simulink model "ClimaMgrForVentAndFlrDistbn"  (R2022b)
settings: Solver=FixedStepDiscrete  StartTime=0.0  StopTime=10.0  FixedStep=1  RelTol=1e-3
(part 2 of 2)

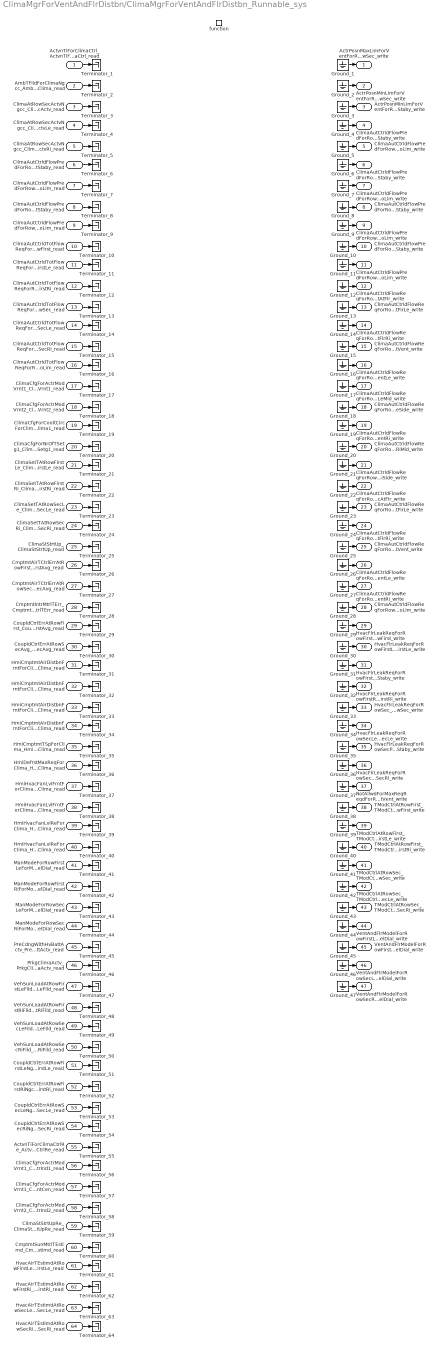
== system ClimaMgrForVentAndFlrDistbn/ClimaMgrForVentAndFlrDistbn_Runnable_sys ==
  ActvnTiForClimaCtrl_ActvnTiForClimaCtrl_read : Inport
  AmbTFildForClimaNgcc_AmbTFildForClima_read : Inport  Port=2
  ClimaAtRowSecActvNgcc_ClimaAtRowSecActv_read : Inport  Port=3
  ClimaAtRowSecActvNgcc_ClimaAtRowSecActvLe_read : Inport  Port=4
  ClimaAtRowSecActvNgcc_ClimaAtRowSecActvRi_read : Inport  Port=5
  ClimaAutCtrldFlowPredForRowFirstAtStaby_ClimaAutCtrldFlowPredForRowFirstAtStaby_read : Inport  Port=6
  ClimaAutCtrldFlowPredForRowFirstNoLim_ClimaAutCtrldFlowPredForRowFirstNoLim_read : Inport  Port=7
  ClimaAutCtrldFlowPredForRowSecAtStaby_ClimaAutCtrldFlowPredForRowSecAtStaby_read : Inport  Port=8
  ClimaAutCtrldFlowPredForRowSecNoLim_ClimaAutCtrldFlowPredForRowSecNoLim_read : Inport  Port=9
  ClimaAutCtrldTotFlowReqForRowFirst_ClimaAutCtrldTotFlowReqForRowFirst_read : Inport  Port=10
  ClimaAutCtrldTotFlowReqForRowFirst_ClimaAutCtrldTotFlowReqForRowFirstLe_read : Inport  Port=11
  ClimaAutCtrldTotFlowReqForRowFirst_ClimaAutCtrldTotFlowReqForRowFirstRi_read : Inport  Port=12
  ClimaAutCtrldTotFlowReqForRowSec_ClimaAutCtrldTotFlowReqForRowSec_read : Inport  Port=13
  ClimaAutCtrldTotFlowReqForRowSec_ClimaAutCtrldTotFlowReqForRowSecLe_read : Inport  Port=14
  ClimaAutCtrldTotFlowReqForRowSec_ClimaAutCtrldTotFlowReqForRowSecRi_read : Inport  Port=15
  ClimaAutCtrldTotFlowReqForRowSecNoLim_ClimaAutCtrldTotFlowReqForRowSecNoLim_read : Inport  Port=16
  ClimaCfgForActrModVrnt1_ClimaCfgForActrModVrnt1_read : Inport  Port=17
  ClimaCfgForActrModVrnt2_ClimaCfgForActrModVrnt2_read : Inport  Port=18
  ClimaCfgForCooltCircForClima1_ClimaCfgForCooltCircForClima1_read : Inport  Port=19
  ClimaCfgForNrOfTSetg1_ClimaCfgForNrOfTSetg1_read : Inport  Port=20
  ClimaSetTAtRowFirstLe_ClimaSetTAtRowFirstLe_read : Inport  Port=21
  ClimaSetTAtRowFirstRi_ClimaSetTAtRowFirstRi_read : Inport  Port=22
  ClimaSetTAtRowSecLe_ClimaSetTAtRowSecLe_read : Inport  Port=23
  ClimaSetTAtRowSecRi_ClimaSetTAtRowSecRi_read : Inport  Port=24
  ClimaStStrtUp_ClimaStStrtUp_read : Inport  Port=25
  CmptmtAirTCtrlErrAtRowFirstAvg_CmptmtAirTCtrlErrAtRowFirstAvg_read : Inport  Port=26
  CmptmtAirTCtrlErrAtRowSecAvg_CmptmtAirTCtrlErrAtRowSecAvg_read : Inport  Port=27
  CmptmtIntrMtrlTErr_CmptmtIntrMtrlTErr_read : Inport  Port=28
  CoupldCtrlErrAtRowFirst_CoupldCtrlErrAtRowFirstAvg_read : Inport  Port=29
  CoupldCtrlErrAtRowSecAvg_CoupldCtrlErrAtRowSecAvg_read : Inport  Port=30
  HmiCmptmtAirDistbnFrntForClima_HmiCmptmtAirDistbnFrntForClima_read : Inport  Port=31
  HmiCmptmtAirDistbnFrntForClima_HmiCmptmtAirDistbnFrntRiForClima_read : Inport  Port=32
  HmiCmptmtAirDistbnFrntForClima_HmiCmptmtAirDistbnReForClima_read : Inport  Port=33
  HmiCmptmtAirDistbnFrntForClima_HmiCmptmtAirDistbnReRiForClima_read : Inport  Port=34
  HmiCmptmtTSpForClima_HmiCmptmtTSpForClima_read : Inport  Port=35
  HmiDefrstMaxReqForClima_HmiDefrstMaxReqForClima_read : Inport  Port=36
  HmiHvacFanLvlFrntForClima_HmiHvacFanLvlFrntForClima_read : Inport  Port=37
  HmiHvacFanLvlFrntForClima_HmiHvacFanLvlFrntRiForClima_read : Inport  Port=38
  HmiHvacFanLvlReForClima_HmiHvacFanLvlReForClima_read : Inport  Port=39
  HmiHvacFanLvlReForClima_HmiHvacFanLvlReRiForClima_read : Inport  Port=40
  ManModeForRowFirstLeForModelDial_ManModeForRowFirstLeForModelDial_read : Inport  Port=41
  ManModeForRowFirstRiForModelDial_ManModeForRowFirstRiForModelDial_read : Inport  Port=42
  ManModeForRowSecLeForModelDial_ManModeForRowSecLeForModelDial_read : Inport  Port=43
  ManModeForRowSecRiForModelDial_ManModeForRowSecRiForModelDial_read : Inport  Port=44
  PreCdngWithHvBattActv_PreCdngWithHvBattActv_read : Inport  Port=45
  PrkgClimaActv_PrkgClimaActv_read : Inport  Port=46
  VehSunLoadAtRowFirstLeFild_VehSunLoadAtRowFirstLeFild_read : Inport  Port=47
  VehSunLoadAtRowFirstRiFild_VehSunLoadAtRowFirstRiFild_read : Inport  Port=48
  VehSunLoadAtRowSecLeFild_VehSunLoadAtRowSecLeFild_read : Inport  Port=49
  VehSunLoadAtRowSecRiFild_VehSunLoadAtRowSecRiFild_read : Inport  Port=50
  CoupldCtrlErrAtRowFirstLeNgcc_CoupldCtrlErrAtRowFirstLe_read : Inport  Port=51
  CoupldCtrlErrAtRowFirstRiNgcc_CoupldCtrlErrAtRowFirstRi_read : Inport  Port=52
  CoupldCtrlErrAtRowSecLeNgcc_CoupldCtrlErrAtRowSecLe_read : Inport  Port=53
  CoupldCtrlErrAtRowSecRiNgcc_CoupldCtrlErrAtRowSecRi_read : Inport  Port=54
  ActvnTiForClimaCtrlRe_ActvnTiForClimaCtrlRe_read : Inport  Port=55
  ClimaCfgForActrModVrnt1_ClimaCfgForActrInd1_read : Inport  Port=56
  ClimaCfgForActrModVrnt1_ClimaCfgForActrModVrntCen_read : Inport  Port=57
  ClimaCfgForActrModVrnt2_ClimaCfgForActrInd2_read : Inport  Port=58
  ClimaStStrtUpRe_ClimaStStrtUpRe_read : Inport  Port=59
  CmptmtSunMtrlTEstimd_CmptmtSunMtrlTEstimd_read : Inport  Port=60
  HvacAirTEstimdAtRowFirstLe_HvacAirTEstimdAtRowFirstLe_read : Inport  Port=61
  HvacAirTEstimdAtRowFirstRi_HvacAirTEstimdAtRowFirstRi_read : Inport  Port=62
  HvacAirTEstimdAtRowSecLe_HvacAirTEstimdAtRowSecLe_read : Inport  Port=63
  HvacAirTEstimdAtRowSecRi_HvacAirTEstimdAtRowSecRi_read : Inport  Port=64
  function : TriggerPort  TriggerType=function-call  VariantControl=(inherit)  SampleTimeType=periodic  SampleTime=-1
  Ground_{1..47} (47 alike) : Ground
  Terminator_{1..64} (64 alike) : Terminator
  ActrPosnMaxLimForVentForRowSec_ActrPosnMaxLimForVentForRowSec_write : Outport
      settings: EnsureOutportIsVirtual=on
  ActrPosnMinLimForVentForRowSec_ActrPosnMinLimForVentForRowSec_write : Outport  Port=2
      settings: EnsureOutportIsVirtual=on
  ActrPosnMinLimForVentForRowSecPredForStaby_ActrPosnMinLimForVentForRowSecPredForStaby_write : Outport  Port=3
      settings: EnsureOutportIsVirtual=on
  ClimaAutCtrldFlowPredForRowFirstAtFlrAtStaby_ClimaAutCtrldFlowPredForRowFirstAtFlrAtStaby_write : Outport  Port=4
      settings: EnsureOutportIsVirtual=on
  ClimaAutCtrldFlowPredForRowFirstAtFlrNoLim_ClimaAutCtrldFlowPredForRowFirstAtFlrNoLim_write : Outport  Port=5
      settings: EnsureOutportIsVirtual=on
  ClimaAutCtrldFlowPredForRowFirstAtVentAtStaby_ClimaAutCtrldFlowPredForRowFirstAtVentAtStaby_write : Outport  Port=6
      settings: EnsureOutportIsVirtual=on
  ClimaAutCtrldFlowPredForRowFirstAtVentNoLim_ClimaAutCtrldFlowPredForRowFirstAtVentNoLim_write : Outport  Port=7
      settings: EnsureOutportIsVirtual=on
  ClimaAutCtrldFlowPredForRowSecAtFlrAtStaby_ClimaAutCtrldFlowPredForRowSecAtFlrAtStaby_write : Outport  Port=8
      settings: EnsureOutportIsVirtual=on
  ClimaAutCtrldFlowPredForRowSecAtFlrNoLim_ClimaAutCtrldFlowPredForRowSecAtFlrNoLim_write : Outport  Port=9
      settings: EnsureOutportIsVirtual=on
  ClimaAutCtrldFlowPredForRowSecAtVentAtStaby_ClimaAutCtrldFlowPredForRowSecAtVentAtStaby_write : Outport  Port=10
      settings: EnsureOutportIsVirtual=on
  ClimaAutCtrldFlowPredForRowSecAtVentNoLim_ClimaAutCtrldFlowPredForRowSecAtVentNoLim_write : Outport  Port=11
      settings: EnsureOutportIsVirtual=on
  ClimaAutCtrldFlowReqForRowFirstAtFlr_ClimaAutCtrldFlowReqForRowFirstAtFlr_write : Outport  Port=12
      settings: EnsureOutportIsVirtual=on
  ClimaAutCtrldFlowReqForRowFirstAtFlr_ClimaAutCtrldFlowReqForRowFirstAtFlrLe_write : Outport  Port=13
      settings: EnsureOutportIsVirtual=on
  ClimaAutCtrldFlowReqForRowFirstAtFlr_ClimaAutCtrldFlowReqForRowFirstAtFlrRi_write : Outport  Port=14
      settings: EnsureOutportIsVirtual=on
  ClimaAutCtrldFlowReqForRowFirstAtVent_ClimaAutCtrldFlowReqForRowFirstAtVent_write : Outport  Port=15
      settings: EnsureOutportIsVirtual=on
  ClimaAutCtrldFlowReqForRowFirstAtVent_ClimaAutCtrldFlowReqForRowFirstAtVentLe_write : Outport  Port=16
      settings: EnsureOutportIsVirtual=on
  ClimaAutCtrldFlowReqForRowFirstAtVent_ClimaAutCtrldFlowReqForRowFirstAtVentLeMid_write : Outport  Port=17
      settings: EnsureOutportIsVirtual=on
  ClimaAutCtrldFlowReqForRowFirstAtVent_ClimaAutCtrldFlowReqForRowFirstAtVentLeSide_write : Outport  Port=18
      settings: EnsureOutportIsVirtual=on
  ClimaAutCtrldFlowReqForRowFirstAtVent_ClimaAutCtrldFlowReqForRowFirstAtVentRi_write : Outport  Port=19
      settings: EnsureOutportIsVirtual=on
  ClimaAutCtrldFlowReqForRowFirstAtVent_ClimaAutCtrldFlowReqForRowFirstAtVentRiMid_write : Outport  Port=20
      settings: EnsureOutportIsVirtual=on
  ClimaAutCtrldFlowReqForRowFirstAtVent_ClimaAutCtrldFlowReqForRowFirstAtVentRiSide_write : Outport  Port=21
      settings: EnsureOutportIsVirtual=on
  ClimaAutCtrldFlowReqForRowSecAtFlr_ClimaAutCtrldFlowReqForRowSecAtFlr_write : Outport  Port=22
      settings: EnsureOutportIsVirtual=on
  ClimaAutCtrldFlowReqForRowSecAtFlr_ClimaAutCtrldFlowReqForRowSecAtFlrLe_write : Outport  Port=23
      settings: EnsureOutportIsVirtual=on
  ClimaAutCtrldFlowReqForRowSecAtFlr_ClimaAutCtrldFlowReqForRowSecAtFlrRi_write : Outport  Port=24
      settings: EnsureOutportIsVirtual=on
  ClimaAutCtrldFlowReqForRowSecAtVent_ClimaAutCtrldFlowReqForRowSecAtVent_write : Outport  Port=25
      settings: EnsureOutportIsVirtual=on
  ClimaAutCtrldFlowReqForRowSecAtVent_ClimaAutCtrldFlowReqForRowSecAtVentLe_write : Outport  Port=26
      settings: EnsureOutportIsVirtual=on
  ClimaAutCtrldFlowReqForRowSecAtVent_ClimaAutCtrldFlowReqForRowSecAtVentRi_write : Outport  Port=27
      settings: EnsureOutportIsVirtual=on
  ClimaAutCtrldFlowReqForRowSecAtVentNoLim_ClimaAutCtrldFlowReqForRowSecAtVentNoLim_write : Outport  Port=28
      settings: EnsureOutportIsVirtual=on
  HvacFlrLeakReqForRowFirst_HvacFlrLeakReqForRowFirst_write : Outport  Port=29
      settings: EnsureOutportIsVirtual=on
  HvacFlrLeakReqForRowFirstLe_HvacFlrLeakReqForRowFirstLe_write : Outport  Port=30
      settings: EnsureOutportIsVirtual=on
  HvacFlrLeakReqForRowFirstPredForStaby_HvacFlrLeakReqForRowFirstPredForStaby_write : Outport  Port=31
      settings: EnsureOutportIsVirtual=on
  HvacFlrLeakReqForRowFirstRi_HvacFlrLeakReqForRowFirstRi_write : Outport  Port=32
      settings: EnsureOutportIsVirtual=on
  HvacFlrLeakReqForRowSec_HvacFlrLeakReqForRowSec_write : Outport  Port=33
      settings: EnsureOutportIsVirtual=on
  HvacFlrLeakReqForRowSecLe_HvacFlrLeakReqForRowSecLe_write : Outport  Port=34
      settings: EnsureOutportIsVirtual=on
  HvacFlrLeakReqForRowSecPredForStaby_HvacFlrLeakReqForRowSecPredForStaby_write : Outport  Port=35
      settings: EnsureOutportIsVirtual=on
  HvacFlrLeakReqForRowSecRi_HvacFlrLeakReqForRowSecRi_write : Outport  Port=36
      settings: EnsureOutportIsVirtual=on
  NotAllwdForMaxReqReqdForRowSecAtVent_NotAllwdForMaxReqReqdForRowSecAtVent_write : Outport  Port=37
      settings: EnsureOutportIsVirtual=on
  TModCtrlAtRowFirst_TModCtrlAtRowFirst_write : Outport  Port=38
      settings: EnsureOutportIsVirtual=on
  TModCtrlAtRowFirst_TModCtrlAtRowFirstLe_write : Outport  Port=39
      settings: EnsureOutportIsVirtual=on
  TModCtrlAtRowFirst_TModCtrlAtRowFirstRi_write : Outport  Port=40
      settings: EnsureOutportIsVirtual=on
  TModCtrlAtRowSec_TModCtrlAtRowSec_write : Outport  Port=41
      settings: EnsureOutportIsVirtual=on
  TModCtrlAtRowSec_TModCtrlAtRowSecLe_write : Outport  Port=42
      settings: EnsureOutportIsVirtual=on
  TModCtrlAtRowSec_TModCtrlAtRowSecRi_write : Outport  Port=43
      settings: EnsureOutportIsVirtual=on
  VentAndFlrModelForRowFirstLeForModelDial_VentAndFlrModelForRowFirstLeForModelDial_write : Outport  Port=44
      settings: EnsureOutportIsVirtual=on
  VentAndFlrModelForRowFirstRiForModelDial_VentAndFlrModelForRowFirstRiForModelDial_write : Outport  Port=45
      settings: EnsureOutportIsVirtual=on
  VentAndFlrModelForRowSecLeForModelDial_VentAndFlrModelForRowSecLeForModelDial_write : Outport  Port=46
      settings: EnsureOutportIsVirtual=on
  VentAndFlrModelForRowSecRiForModelDial_VentAndFlrModelForRowSecRiForModelDial_write : Outport  Port=47
      settings: EnsureOutportIsVirtual=on
  wires:
  ActvnTiForClimaCtrl_ActvnTiForClimaCtrl_read/1 -> Terminator_1/1
  AmbTFildForClimaNgcc_AmbTFildForClima_read/1 -> Terminator_2/1
  ClimaAtRowSecActvNgcc_ClimaAtRowSecActv_read/1 -> Terminator_3/1
  ClimaAtRowSecActvNgcc_ClimaAtRowSecActvLe_read/1 -> Terminator_4/1
  ClimaAtRowSecActvNgcc_ClimaAtRowSecActvRi_read/1 -> Terminator_5/1
  ClimaAutCtrldFlowPredForRowFirstAtStaby_ClimaAutCtrldFlowPredForRowFirstAtStaby_read/1 -> Terminator_6/1
  ClimaAutCtrldFlowPredForRowFirstNoLim_ClimaAutCtrldFlowPredForRowFirstNoLim_read/1 -> Terminator_7/1
  ClimaAutCtrldFlowPredForRowSecAtStaby_ClimaAutCtrldFlowPredForRowSecAtStaby_read/1 -> Terminator_8/1
  ClimaAutCtrldFlowPredForRowSecNoLim_ClimaAutCtrldFlowPredForRowSecNoLim_read/1 -> Terminator_9/1
  ClimaAutCtrldTotFlowReqForRowFirst_ClimaAutCtrldTotFlowReqForRowFirst_read/1 -> Terminator_10/1
  ClimaAutCtrldTotFlowReqForRowFirst_ClimaAutCtrldTotFlowReqForRowFirstLe_read/1 -> Terminator_11/1
  ClimaAutCtrldTotFlowReqForRowFirst_ClimaAutCtrldTotFlowReqForRowFirstRi_read/1 -> Terminator_12/1
  ClimaAutCtrldTotFlowReqForRowSec_ClimaAutCtrldTotFlowReqForRowSec_read/1 -> Terminator_13/1
  ClimaAutCtrldTotFlowReqForRowSec_ClimaAutCtrldTotFlowReqForRowSecLe_read/1 -> Terminator_14/1
  ClimaAutCtrldTotFlowReqForRowSec_ClimaAutCtrldTotFlowReqForRowSecRi_read/1 -> Terminator_15/1
  ClimaAutCtrldTotFlowReqForRowSecNoLim_ClimaAutCtrldTotFlowReqForRowSecNoLim_read/1 -> Terminator_16/1
  ClimaCfgForActrModVrnt1_ClimaCfgForActrModVrnt1_read/1 -> Terminator_17/1
  ClimaCfgForActrModVrnt2_ClimaCfgForActrModVrnt2_read/1 -> Terminator_18/1
  ClimaCfgForCooltCircForClima1_ClimaCfgForCooltCircForClima1_read/1 -> Terminator_19/1
  ClimaCfgForNrOfTSetg1_ClimaCfgForNrOfTSetg1_read/1 -> Terminator_20/1
  ClimaSetTAtRowFirstLe_ClimaSetTAtRowFirstLe_read/1 -> Terminator_21/1
  ClimaSetTAtRowFirstRi_ClimaSetTAtRowFirstRi_read/1 -> Terminator_22/1
  ClimaSetTAtRowSecLe_ClimaSetTAtRowSecLe_read/1 -> Terminator_23/1
  ClimaSetTAtRowSecRi_ClimaSetTAtRowSecRi_read/1 -> Terminator_24/1
  ClimaStStrtUp_ClimaStStrtUp_read/1 -> Terminator_25/1
  CmptmtAirTCtrlErrAtRowFirstAvg_CmptmtAirTCtrlErrAtRowFirstAvg_read/1 -> Terminator_26/1
  CmptmtAirTCtrlErrAtRowSecAvg_CmptmtAirTCtrlErrAtRowSecAvg_read/1 -> Terminator_27/1
  CmptmtIntrMtrlTErr_CmptmtIntrMtrlTErr_read/1 -> Terminator_28/1
  CoupldCtrlErrAtRowFirst_CoupldCtrlErrAtRowFirstAvg_read/1 -> Terminator_29/1
  CoupldCtrlErrAtRowSecAvg_CoupldCtrlErrAtRowSecAvg_read/1 -> Terminator_30/1
  HmiCmptmtAirDistbnFrntForClima_HmiCmptmtAirDistbnFrntForClima_read/1 -> Terminator_31/1
  HmiCmptmtAirDistbnFrntForClima_HmiCmptmtAirDistbnFrntRiForClima_read/1 -> Terminator_32/1
  HmiCmptmtAirDistbnFrntForClima_HmiCmptmtAirDistbnReForClima_read/1 -> Terminator_33/1
  HmiCmptmtAirDistbnFrntForClima_HmiCmptmtAirDistbnReRiForClima_read/1 -> Terminator_34/1
  HmiCmptmtTSpForClima_HmiCmptmtTSpForClima_read/1 -> Terminator_35/1
  HmiDefrstMaxReqForClima_HmiDefrstMaxReqForClima_read/1 -> Terminator_36/1
  HmiHvacFanLvlFrntForClima_HmiHvacFanLvlFrntForClima_read/1 -> Terminator_37/1
  HmiHvacFanLvlFrntForClima_HmiHvacFanLvlFrntRiForClima_read/1 -> Terminator_38/1
  HmiHvacFanLvlReForClima_HmiHvacFanLvlReForClima_read/1 -> Terminator_39/1
  HmiHvacFanLvlReForClima_HmiHvacFanLvlReRiForClima_read/1 -> Terminator_40/1
  ManModeForRowFirstLeForModelDial_ManModeForRowFirstLeForModelDial_read/1 -> Terminator_41/1
  ManModeForRowFirstRiForModelDial_ManModeForRowFirstRiForModelDial_read/1 -> Terminator_42/1
  ManModeForRowSecLeForModelDial_ManModeForRowSecLeForModelDial_read/1 -> Terminator_43/1
  ManModeForRowSecRiForModelDial_ManModeForRowSecRiForModelDial_read/1 -> Terminator_44/1
  PreCdngWithHvBattActv_PreCdngWithHvBattActv_read/1 -> Terminator_45/1
  PrkgClimaActv_PrkgClimaActv_read/1 -> Terminator_46/1
  VehSunLoadAtRowFirstLeFild_VehSunLoadAtRowFirstLeFild_read/1 -> Terminator_47/1
  VehSunLoadAtRowFirstRiFild_VehSunLoadAtRowFirstRiFild_read/1 -> Terminator_48/1
  VehSunLoadAtRowSecLeFild_VehSunLoadAtRowSecLeFild_read/1 -> Terminator_49/1
  VehSunLoadAtRowSecRiFild_VehSunLoadAtRowSecRiFild_read/1 -> Terminator_50/1
  CoupldCtrlErrAtRowFirstLeNgcc_CoupldCtrlErrAtRowFirstLe_read/1 -> Terminator_51/1
  CoupldCtrlErrAtRowFirstRiNgcc_CoupldCtrlErrAtRowFirstRi_read/1 -> Terminator_52/1
  CoupldCtrlErrAtRowSecLeNgcc_CoupldCtrlErrAtRowSecLe_read/1 -> Terminator_53/1
  CoupldCtrlErrAtRowSecRiNgcc_CoupldCtrlErrAtRowSecRi_read/1 -> Terminator_54/1
  ActvnTiForClimaCtrlRe_ActvnTiForClimaCtrlRe_read/1 -> Terminator_55/1
  ClimaCfgForActrModVrnt1_ClimaCfgForActrInd1_read/1 -> Terminator_56/1
  ClimaCfgForActrModVrnt1_ClimaCfgForActrModVrntCen_read/1 -> Terminator_57/1
  ClimaCfgForActrModVrnt2_ClimaCfgForActrInd2_read/1 -> Terminator_58/1
  ClimaStStrtUpRe_ClimaStStrtUpRe_read/1 -> Terminator_59/1
  CmptmtSunMtrlTEstimd_CmptmtSunMtrlTEstimd_read/1 -> Terminator_60/1
  HvacAirTEstimdAtRowFirstLe_HvacAirTEstimdAtRowFirstLe_read/1 -> Terminator_61/1
  HvacAirTEstimdAtRowFirstRi_HvacAirTEstimdAtRowFirstRi_read/1 -> Terminator_62/1
  HvacAirTEstimdAtRowSecLe_HvacAirTEstimdAtRowSecLe_read/1 -> Terminator_63/1
  HvacAirTEstimdAtRowSecRi_HvacAirTEstimdAtRowSecRi_read/1 -> Terminator_64/1
  Ground_1/1 -> ActrPosnMaxLimForVentForRowSec_ActrPosnMaxLimForVentForRowSec_write/1
  Ground_2/1 -> ActrPosnMinLimForVentForRowSec_ActrPosnMinLimForVentForRowSec_write/1
  Ground_3/1 -> ActrPosnMinLimForVentForRowSecPredForStaby_ActrPosnMinLimForVentForRowSecPredForStaby_write/1
  Ground_4/1 -> ClimaAutCtrldFlowPredForRowFirstAtFlrAtStaby_ClimaAutCtrldFlowPredForRowFirstAtFlrAtStaby_write/1
  Ground_5/1 -> ClimaAutCtrldFlowPredForRowFirstAtFlrNoLim_ClimaAutCtrldFlowPredForRowFirstAtFlrNoLim_write/1
  Ground_6/1 -> ClimaAutCtrldFlowPredForRowFirstAtVentAtStaby_ClimaAutCtrldFlowPredForRowFirstAtVentAtStaby_write/1
  Ground_7/1 -> ClimaAutCtrldFlowPredForRowFirstAtVentNoLim_ClimaAutCtrldFlowPredForRowFirstAtVentNoLim_write/1
  Ground_8/1 -> ClimaAutCtrldFlowPredForRowSecAtFlrAtStaby_ClimaAutCtrldFlowPredForRowSecAtFlrAtStaby_write/1
  Ground_9/1 -> ClimaAutCtrldFlowPredForRowSecAtFlrNoLim_ClimaAutCtrldFlowPredForRowSecAtFlrNoLim_write/1
  Ground_10/1 -> ClimaAutCtrldFlowPredForRowSecAtVentAtStaby_ClimaAutCtrldFlowPredForRowSecAtVentAtStaby_write/1
  Ground_11/1 -> ClimaAutCtrldFlowPredForRowSecAtVentNoLim_ClimaAutCtrldFlowPredForRowSecAtVentNoLim_write/1
  Ground_12/1 -> ClimaAutCtrldFlowReqForRowFirstAtFlr_ClimaAutCtrldFlowReqForRowFirstAtFlr_write/1
  Ground_13/1 -> ClimaAutCtrldFlowReqForRowFirstAtFlr_ClimaAutCtrldFlowReqForRowFirstAtFlrLe_write/1
  Ground_14/1 -> ClimaAutCtrldFlowReqForRowFirstAtFlr_ClimaAutCtrldFlowReqForRowFirstAtFlrRi_write/1
  Ground_15/1 -> ClimaAutCtrldFlowReqForRowFirstAtVent_ClimaAutCtrldFlowReqForRowFirstAtVent_write/1
  Ground_16/1 -> ClimaAutCtrldFlowReqForRowFirstAtVent_ClimaAutCtrldFlowReqForRowFirstAtVentLe_write/1
  Ground_17/1 -> ClimaAutCtrldFlowReqForRowFirstAtVent_ClimaAutCtrldFlowReqForRowFirstAtVentLeMid_write/1
  Ground_18/1 -> ClimaAutCtrldFlowReqForRowFirstAtVent_ClimaAutCtrldFlowReqForRowFirstAtVentLeSide_write/1
  Ground_19/1 -> ClimaAutCtrldFlowReqForRowFirstAtVent_ClimaAutCtrldFlowReqForRowFirstAtVentRi_write/1
  Ground_20/1 -> ClimaAutCtrldFlowReqForRowFirstAtVent_ClimaAutCtrldFlowReqForRowFirstAtVentRiMid_write/1
  Ground_21/1 -> ClimaAutCtrldFlowReqForRowFirstAtVent_ClimaAutCtrldFlowReqForRowFirstAtVentRiSide_write/1
  Ground_22/1 -> ClimaAutCtrldFlowReqForRowSecAtFlr_ClimaAutCtrldFlowReqForRowSecAtFlr_write/1
  Ground_23/1 -> ClimaAutCtrldFlowReqForRowSecAtFlr_ClimaAutCtrldFlowReqForRowSecAtFlrLe_write/1
  Ground_24/1 -> ClimaAutCtrldFlowReqForRowSecAtFlr_ClimaAutCtrldFlowReqForRowSecAtFlrRi_write/1
  Ground_25/1 -> ClimaAutCtrldFlowReqForRowSecAtVent_ClimaAutCtrldFlowReqForRowSecAtVent_write/1
  Ground_26/1 -> ClimaAutCtrldFlowReqForRowSecAtVent_ClimaAutCtrldFlowReqForRowSecAtVentLe_write/1
  Ground_27/1 -> ClimaAutCtrldFlowReqForRowSecAtVent_ClimaAutCtrldFlowReqForRowSecAtVentRi_write/1
  Ground_28/1 -> ClimaAutCtrldFlowReqForRowSecAtVentNoLim_ClimaAutCtrldFlowReqForRowSecAtVentNoLim_write/1
  Ground_29/1 -> HvacFlrLeakReqForRowFirst_HvacFlrLeakReqForRowFirst_write/1
  Ground_30/1 -> HvacFlrLeakReqForRowFirstLe_HvacFlrLeakReqForRowFirstLe_write/1
  Ground_31/1 -> HvacFlrLeakReqForRowFirstPredForStaby_HvacFlrLeakReqForRowFirstPredForStaby_write/1
  Ground_32/1 -> HvacFlrLeakReqForRowFirstRi_HvacFlrLeakReqForRowFirstRi_write/1
  Ground_33/1 -> HvacFlrLeakReqForRowSec_HvacFlrLeakReqForRowSec_write/1
  Ground_34/1 -> HvacFlrLeakReqForRowSecLe_HvacFlrLeakReqForRowSecLe_write/1
  Ground_35/1 -> HvacFlrLeakReqForRowSecPredForStaby_HvacFlrLeakReqForRowSecPredForStaby_write/1
  Ground_36/1 -> HvacFlrLeakReqForRowSecRi_HvacFlrLeakReqForRowSecRi_write/1
  Ground_37/1 -> NotAllwdForMaxReqReqdForRowSecAtVent_NotAllwdForMaxReqReqdForRowSecAtVent_write/1
  Ground_38/1 -> TModCtrlAtRowFirst_TModCtrlAtRowFirst_write/1
  Ground_39/1 -> TModCtrlAtRowFirst_TModCtrlAtRowFirstLe_write/1
  Ground_40/1 -> TModCtrlAtRowFirst_TModCtrlAtRowFirstRi_write/1
  Ground_41/1 -> TModCtrlAtRowSec_TModCtrlAtRowSec_write/1
  Ground_42/1 -> TModCtrlAtRowSec_TModCtrlAtRowSecLe_write/1
  Ground_43/1 -> TModCtrlAtRowSec_TModCtrlAtRowSecRi_write/1
  Ground_44/1 -> VentAndFlrModelForRowFirstLeForModelDial_VentAndFlrModelForRowFirstLeForModelDial_write/1
  Ground_45/1 -> VentAndFlrModelForRowFirstRiForModelDial_VentAndFlrModelForRowFirstRiForModelDial_write/1
  Ground_46/1 -> VentAndFlrModelForRowSecLeForModelDial_VentAndFlrModelForRowSecLeForModelDial_write/1
  Ground_47/1 -> VentAndFlrModelForRowSecRiForModelDial_VentAndFlrModelForRowSecRiForModelDial_write/1
